simulink model "Complete_Model"  (R2023a)
settings: Solver=VariableStepAuto  StartTime=0.0  StopTime=22  MinStep=5e-5  RelTol=1e-3
values from neighbouring files (symbols this model uses):
  Cbus = 80e-3*n_wt_agg*u^2    (= 1.33333333333)    [param.m]
  D = 1e-3    (= 0.001)    [param.m]
  f = 50    [param.m]
  Fs = Vs * sqrt(2/3)/(2*pi*f)    [param.m]
  fsw = 4e3    (= 4000)    [param.m]
  Idr_rated = sqrt(2)*Is    [param.m]
  J = 127*n_wt_agg    (= 19050)    [param.m]
  ki_id = (wni^2)*Lr*sigma    (= 3.27733001332)    [param.m]
  ki_idg = (wnig^2)*Lg    (= 0.263189450696)    [param.m]
  ki_iq = ki_id    (= 3.27733001332)    [param.m]
  ki_iqg = ki_idg    (= 0.263189450696)    [param.m]
  ki_v = -300000*n_wt_agg    (= -45000000)    [param.m]
  Kopt = ((0.5*rho*pi*(Radius^5)*Cp_max)/(lambda_opt^3))    (= 296454.198186)    [param.m]
  kp_id = (2*wni*sigma*Lr) - Rr    (= 0.00384733333333)    [param.m]
  kp_idg = ((2*wnig*Lg)-Rg)    (= 0.00167538274858)    [param.m]
  kp_iq = kp_id    (= 0.00384733333333)    [param.m]
  kp_iqg = kp_idg    (= 0.00167538274858)    [param.m]
  kp_v = -1000*n_wt_agg    (= -150000)    [param.m]
  Kpg = 1/(1.5*Vr*sqrt(2/3))    [param.m]
  Kqg = -Kpg    [param.m]
  lambda_opt = 7.2    [param.m]
  Lg = 400e-6 /n_wt_agg    (= 2.66666666667e-06)    [param.m]
  Lm = 2.5e-3 /n_wt_agg    (= 1.66666666667e-05)    [param.m]
  Lr = Lm + Lsi    (= 1.72466666667e-05)    [param.m]
  Ls = Lm + Lsi    (= 1.72466666667e-05)    [param.m]
  Lsi = 0.087e-3 /n_wt_agg    (= 5.8e-07)    [param.m]
  N = 100    [param.m]
  n_wt_agg = 150    [param.m]
  p = 2    [param.m]
  Ps = 2e6*n_wt_agg    (= 300000000)    [param.m]
  Radius = 42    [param.m]
  Rg = 20e-6 /n_wt_agg    (= 1.33333333333e-07)    [param.m]
  rho = 1.225    [param.m]
  Rr = 2.9e-3 /n_wt_agg    (= 1.93333333333e-05)    [param.m]
  Rs = 2.6e-3 /n_wt_agg    (= 1.73333333333e-05)    [param.m]
  sigma = 1 - Lm^2 / (Ls*Lr)    (= 0.0661284179513)    [param.m]
  smax = 1/3    (= 0.333333333333)    [param.m]
  tab_lambda = [0.1:0.01:11.8]    [param.m]
  Vbus = 1150/u    (= 3450)    [param.m]
  Vs = 690    [param.m]
undefined symbols (not defined in the file or in its folder): Cp, D1, Delta, DiscIntNeverNeededParam, magnitude
libraries: spsSecondOrderFilterLib x133, spsThreePhaseVIMeasurementLib x58, spsPower3phInstantaneousLib x52, simulink x41, spsThreePhaseTransformerTwoWindingsLib x27, spsUniversalBridgeLib x22, spsPWMGenerator2LevelLib x22, spsSeriesRLCBranchLib x19, spsFirstOrderFilterLib x18, spsThreePhasePISectionLineLib x17, spsGroundLib x17, spsThreePhaseSeriesRLCBranchLib x17
(part 11 of 15)

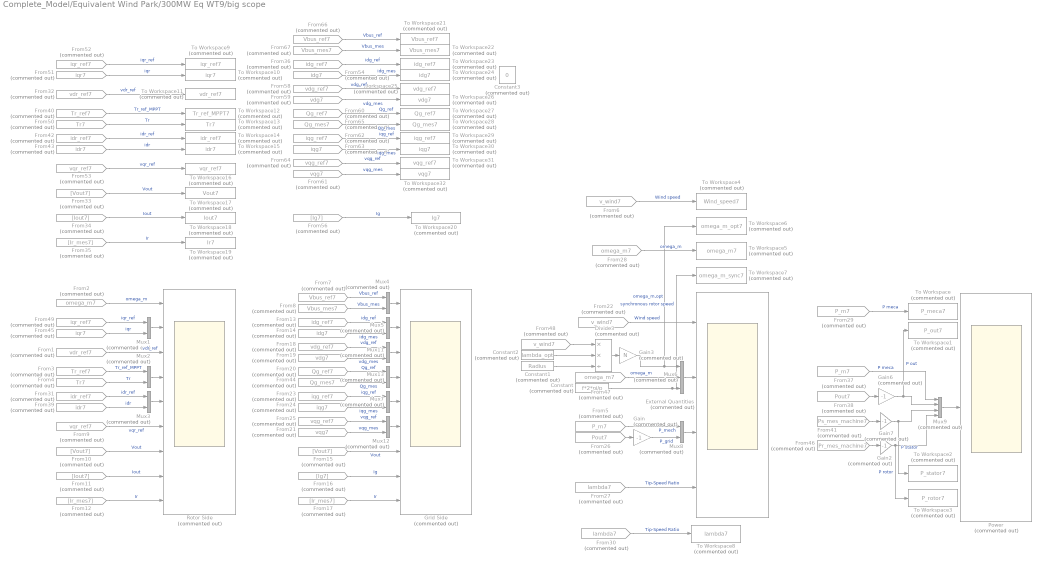
== system Complete_Model/Equivalent Wind Park/300MW Eq WT9/big scope ==
  Constant : Constant  Commented=on  Value=f*2*pi/p
  Constant1 : Constant  Commented=on  Value=Radius
  Constant2 : Constant  Commented=on  Value=lambda_opt
  Constant3 : Constant  Commented=on  Value=0
  Divide3 : Product  Commented=on  Inputs=**/
  External Quantities : Scope  Commented=on  NumInputPorts=4
  From1 : From  Commented=on  GotoTag=vdr_ref7  TagVisibility=global
  From10 : From  Commented=on  GotoTag=Vout7  TagVisibility=global
  From11 : From  Commented=on  GotoTag=Iout7  TagVisibility=global
  From12 : From  Commented=on  GotoTag=Ir_mes7  TagVisibility=global
  From13 : From  Commented=on  GotoTag=idg_ref7  TagVisibility=global
  From14 : From  Commented=on  GotoTag=idg7  TagVisibility=global
  From15 : From  Commented=on  GotoTag=Vout7  TagVisibility=global
  From16 : From  Commented=on  GotoTag=Ig7  TagVisibility=global
  From17 : From  Commented=on  GotoTag=Ir_mes7  TagVisibility=global
  From18 : From  Commented=on  GotoTag=vdg_ref7  TagVisibility=global
  From19 : From  Commented=on  GotoTag=vdg7  TagVisibility=global
  From2 : From  Commented=on  GotoTag=omega_m7  TagVisibility=global
  From20 : From  Commented=on  GotoTag=Qg_ref7  TagVisibility=global
  From21 : From  Commented=on  GotoTag=vqg7  TagVisibility=global
  From22 : From  Commented=on  GotoTag=v_wind7  TagVisibility=global
  From23 : From  Commented=on  GotoTag=iqg_ref7  TagVisibility=global
  From24 : From  Commented=on  GotoTag=iqg7  TagVisibility=global
  From25 : From  Commented=on  GotoTag=vqg_ref7  TagVisibility=global
  From26 : From  Commented=on  GotoTag=Pout7  TagVisibility=global
  From27 : From  Commented=on  GotoTag=lambda7  TagVisibility=global
  From28 : From  Commented=on  GotoTag=omega_m7  TagVisibility=global
  From29 : From  Commented=on  GotoTag=P_m7  TagVisibility=global
  From3 : From  Commented=on  GotoTag=Tr_ref7  TagVisibility=global
  From30 : From  Commented=on  GotoTag=lambda7  TagVisibility=global
  From31 : From  Commented=on  GotoTag=idr_ref7  TagVisibility=global
  From32 : From  Commented=on  GotoTag=vdr_ref7  TagVisibility=global
  From33 : From  Commented=on  GotoTag=Vout7  TagVisibility=global
  From34 : From  Commented=on  GotoTag=Iout7  TagVisibility=global
  From35 : From  Commented=on  GotoTag=Ir_mes7  TagVisibility=global
  From36 : From  Commented=on  GotoTag=idg_ref7  TagVisibility=global
  From37 : From  Commented=on  GotoTag=P_m7  TagVisibility=global
  From38 : From  Commented=on  GotoTag=Pout7  TagVisibility=global
  From39 : From  Commented=on  GotoTag=idr7  TagVisibility=global
  From4 : From  Commented=on  GotoTag=Tr7  TagVisibility=global
  From40 : From  Commented=on  GotoTag=Tr_ref7  TagVisibility=global
  From41 : From  Commented=on  GotoTag=Ps_mes_machine7  TagVisibility=global
  From42 : From  Commented=on  GotoTag=idr_ref7  TagVisibility=global
  From43 : From  Commented=on  GotoTag=idr7  TagVisibility=global
  From44 : From  Commented=on  GotoTag=Qg_mes7  TagVisibility=global
  From45 : From  Commented=on  GotoTag=iqr7  TagVisibility=global
  From46 : From  Commented=on  GotoTag=Pr_mes_machine7  TagVisibility=global
  From47 : From  Commented=on  GotoTag=omega_m7  TagVisibility=global
  From48 : From  Commented=on  GotoTag=v_wind7  TagVisibility=global
  From49 : From  Commented=on  GotoTag=iqr_ref7  TagVisibility=global
  From5 : From  Commented=on  GotoTag=P_m7  TagVisibility=global
  From50 : From  Commented=on  GotoTag=Tr7  TagVisibility=global
  From51 : From  Commented=on  GotoTag=iqr7  TagVisibility=global
  From52 : From  Commented=on  GotoTag=iqr_ref7  TagVisibility=global
  From53 : From  Commented=on  GotoTag=vqr_ref7  TagVisibility=global
  From54 : From  Commented=on  GotoTag=idg7  TagVisibility=global
  From56 : From  Commented=on  GotoTag=Ig7  TagVisibility=global
  From58 : From  Commented=on  GotoTag=vdg_ref7  TagVisibility=global
  From59 : From  Commented=on  GotoTag=vdg7  TagVisibility=global
  From6 : From  Commented=on  GotoTag=v_wind7  TagVisibility=global
  From60 : From  Commented=on  GotoTag=Qg_ref7  TagVisibility=global
  From61 : From  Commented=on  GotoTag=vqg7  TagVisibility=global
  From62 : From  Commented=on  GotoTag=iqg_ref7  TagVisibility=global
  From63 : From  Commented=on  GotoTag=iqg7  TagVisibility=global
  From64 : From  Commented=on  GotoTag=vqg_ref7  TagVisibility=global
  From65 : From  Commented=on  GotoTag=Qg_mes7  TagVisibility=global
  From66 : From  Commented=on  GotoTag=Vbus_ref7  TagVisibility=global
  From67 : From  Commented=on  GotoTag=Vbus_mes7  TagVisibility=global
  From7 : From  Commented=on  GotoTag=Vbus_ref7  TagVisibility=global
  From8 : From  Commented=on  GotoTag=Vbus_mes7  TagVisibility=global
  From9 : From  Commented=on  GotoTag=vqr_ref7  TagVisibility=global
  Gain{,2,6..7} (4 alike) : Gain  Commented=on  Gain=-1
  Gain3 : Gain  Commented=on  Gain=N
  Grid Side : Scope  Commented=on  NumInputPorts=9
  Mux{1..5,7..8,11..13} (10 alike) : Mux  Commented=on  Inputs=2
  Mux6 : Mux  Commented=on  Inputs=3
  Mux9 : Mux  Commented=on
  Power : Scope  Commented=on
  Rotor Side : Scope  Commented=on  NumInputPorts=9
  To Workspace : ToWorkspace  Commented=on  VariableName=P_meca7  SaveFormat=Timeseries  SampleTime=-1
  To Workspace1 : ToWorkspace  Commented=on  VariableName=P_out7  SaveFormat=Timeseries  SampleTime=-1
  To Workspace10 : ToWorkspace  Commented=on  VariableName=iqr7  SaveFormat=Timeseries  SampleTime=-1
  To Workspace11 : ToWorkspace  Commented=on  VariableName=vdr_ref7  SaveFormat=Timeseries  SampleTime=-1
  To Workspace12 : ToWorkspace  Commented=on  VariableName=Tr_ref_MPPT7  SaveFormat=Timeseries  SampleTime=-1
  To Workspace13 : ToWorkspace  Commented=on  VariableName=Tr7  SaveFormat=Timeseries  SampleTime=-1
  To Workspace14 : ToWorkspace  Commented=on  VariableName=idr_ref7  SaveFormat=Timeseries  SampleTime=-1
  To Workspace15 : ToWorkspace  Commented=on  VariableName=idr7  SaveFormat=Timeseries  SampleTime=-1
  To Workspace16 : ToWorkspace  Commented=on  VariableName=vqr_ref7  SaveFormat=Timeseries  SampleTime=-1
  To Workspace17 : ToWorkspace  Commented=on  VariableName=Vout7  SaveFormat=Timeseries  SampleTime=-1
  To Workspace18 : ToWorkspace  Commented=on  VariableName=Iout7  SaveFormat=Timeseries  SampleTime=-1
  To Workspace19 : ToWorkspace  Commented=on  VariableName=Ir7  SaveFormat=Timeseries  SampleTime=-1
  To Workspace2 : ToWorkspace  Commented=on  VariableName=P_stator7  SaveFormat=Timeseries  SampleTime=-1
  To Workspace20 : ToWorkspace  Commented=on  VariableName=Ig7  SaveFormat=Timeseries  SampleTime=-1
  To Workspace21 : ToWorkspace  Commented=on  VariableName=Vbus_ref7  SaveFormat=Timeseries  SampleTime=-1
  To Workspace22 : ToWorkspace  Commented=on  VariableName=Vbus_mes7  SaveFormat=Timeseries  SampleTime=-1
  To Workspace23 : ToWorkspace  Commented=on  VariableName=idg_ref7  SaveFormat=Timeseries  SampleTime=-1
  To Workspace24 : ToWorkspace  Commented=on  VariableName=idg7  SaveFormat=Timeseries  SampleTime=-1
  To Workspace25 : ToWorkspace  Commented=on  VariableName=vdg_ref7  SaveFormat=Timeseries  SampleTime=-1
  To Workspace26 : ToWorkspace  Commented=on  VariableName=vdg7  SaveFormat=Timeseries  SampleTime=-1
  To Workspace27 : ToWorkspace  Commented=on  VariableName=Qg_ref7  SaveFormat=Timeseries  SampleTime=-1
  To Workspace28 : ToWorkspace  Commented=on  VariableName=Qg_mes7  SaveFormat=Timeseries  SampleTime=-1
  To Workspace29 : ToWorkspace  Commented=on  VariableName=iqg_ref7  SaveFormat=Timeseries  SampleTime=-1
  To Workspace3 : ToWorkspace  Commented=on  VariableName=P_rotor7  SaveFormat=Timeseries  SampleTime=-1
  To Workspace30 : ToWorkspace  Commented=on  VariableName=iqg7  SaveFormat=Timeseries  SampleTime=-1
  To Workspace31 : ToWorkspace  Commented=on  VariableName=vqg_ref7  SaveFormat=Timeseries  SampleTime=-1
  To Workspace32 : ToWorkspace  Commented=on  VariableName=vqg7  SaveFormat=Timeseries  SampleTime=-1
  To Workspace4 : ToWorkspace  Commented=on  VariableName=Wind_speed7  SaveFormat=Timeseries  SampleTime=-1
  To Workspace5 : ToWorkspace  Commented=on  VariableName=omega_m7  SaveFormat=Timeseries  SampleTime=-1
  To Workspace6 : ToWorkspace  Commented=on  VariableName=omega_m_opt7  SaveFormat=Timeseries  SampleTime=-1
  To Workspace7 : ToWorkspace  Commented=on  VariableName=omega_m_sync7  SaveFormat=Timeseries  SampleTime=-1
  To Workspace8 : ToWorkspace  Commented=on  VariableName=lambda7  SaveFormat=Timeseries  SampleTime=-1
  To Workspace9 : ToWorkspace  Commented=on  VariableName=iqr_ref7  SaveFormat=Timeseries  SampleTime=-1
  wires:
  From61/1 -> To Workspace32/1  "vqg_mes"
  From64/1 -> To Workspace31/1  "vqg_ref"
  From63/1 -> To Workspace30/1  "iqg_mes"
  From62/1 -> To Workspace29/1  "iqg_ref"
  From65/1 -> To Workspace28/1  "Qg_mes"
  From59/1 -> To Workspace26/1  "vdg_mes"
  From60/1 -> To Workspace27/1  "Qg_ref"
  From58/1 -> To Workspace25/1  "vdg_ref"
  From54/1 -> To Workspace24/1  "idg_mes"
  From36/1 -> To Workspace23/1  "idg_ref"
  From67/1 -> To Workspace22/1  "Vbus_mes"
  From66/1 -> To Workspace21/1  "Vbus_ref"
  From56/1 -> To Workspace20/1  "Ig"
  From35/1 -> To Workspace19/1  "Ir"
  From34/1 -> To Workspace18/1  "Iout"
  From33/1 -> To Workspace17/1  "Vout"
  From53/1 -> To Workspace16/1  "vqr_ref"
  From43/1 -> To Workspace15/1  "idr"
  From42/1 -> To Workspace14/1  "idr_ref"
  From50/1 -> To Workspace13/1  "Tr"
  From40/1 -> To Workspace12/1  "Tr_ref_MPPT"
  From32/1 -> To Workspace11/1  "vdr_ref"
  From51/1 -> To Workspace10/1  "iqr"
  From52/1 -> To Workspace9/1  "iqr_ref"
  From30/1 -> To Workspace8/1  "Tip-Speed Ratio"
  From29/1 -> To Workspace/1  "P meca"
  From28/1 -> To Workspace5/1  "omega_m"
  From6/1 -> To Workspace4/1  "Wind speed"
  From27/1 -> External Quantities/4  "Tip-Speed Ratio"
  Gain3/1 -> Mux6/1, To Workspace6/1  "omega_m,opt"
  Divide3/1 -> Gain3/1
  Constant1/1 -> Divide3/3
  Constant2/1 -> Divide3/2
  From48/1 -> Divide3/1
  From39/1 -> Mux3/2  "idr"
  From4/1 -> Mux2/2  "Tr"
  From1/1 -> Rotor Side/3  "vdr_ref"
  From49/1 -> Mux1/1  "iqr_ref"
  Mux13/1 -> Grid Side/4
  From44/1 -> Mux13/2  "Qg_mes"
  From20/1 -> Mux13/1  "Qg_ref"
  Mux12/1 -> Grid Side/6
  From25/1 -> Mux12/1  "vqg_ref"
  From21/1 -> Mux12/2  "vqg_mes"
  Gain2/1 -> Mux9/4, To Workspace3/1  "P rotor"
  From38/1 -> Gain6/1
  Mux9/1 -> Power/1
  From37/1 -> Mux9/1  "P meca"
  From46/1 -> Gain2/1
  Gain7/1 -> Mux9/3, To Workspace2/1  "P stator"
  From41/1 -> Gain7/1
  Gain6/1 -> Mux9/2, To Workspace1/1  "P out"
  Mux11/1 -> Grid Side/3
  From19/1 -> Mux11/2  "vdg_mes"
  From18/1 -> Mux11/1  "vdg_ref"
  From5/1 -> Mux8/1  "P_mech"
  Constant/1 -> Mux6/3, To Workspace7/1  "synchronous rotor speed"
  From47/1 -> Mux6/2  "omega_m"
  From22/1 -> External Quantities/1  "Wind speed"
  Mux6/1 -> External Quantities/2
  Mux8/1 -> External Quantities/3
  From26/1 -> Gain/1
  Gain/1 -> Mux8/2  "P_grid"
  From2/1 -> Rotor Side/1  "omega_m"
  From13/1 -> Mux5/1  "idg_ref"
  Mux4/1 -> Grid Side/1
  From14/1 -> Mux5/2  "idg_mes"
  Mux5/1 -> Grid Side/2
  From8/1 -> Mux4/2  "Vbus_mes"
  Mux7/1 -> Grid Side/5
  From7/1 -> Mux4/1  "Vbus_ref"
  From24/1 -> Mux7/2  "iqg_mes"
  From17/1 -> Grid Side/9  "Ir"
  From15/1 -> Grid Side/7  "Vout"
  From23/1 -> Mux7/1  "iqg_ref"
  From16/1 -> Grid Side/8  "Ig"
  From12/1 -> Rotor Side/9  "Ir"
  From11/1 -> Rotor Side/8  "Iout"
  From10/1 -> Rotor Side/7  "Vout"
  From9/1 -> Rotor Side/6  "vqr_ref"
  From31/1 -> Mux3/1  "idr_ref"
  Mux3/1 -> Rotor Side/5
  Mux2/1 -> Rotor Side/4
  From3/1 -> Mux2/1  "Tr_ref_MPPT"
  Mux1/1 -> Rotor Side/2
  From45/1 -> Mux1/2  "iqr"

== system Complete_Model/HVDC Link/Grid side control/abc->alpha//beta ==
  abc : Inport
  Fcn1 : Fcn  Expr=2/3 * (u[1]-u[2]/2-u[3]/2)
  Fcn2 : Fcn  Expr=2/3 * (u[2] * (sqrt(3)/2) - u[3]*(sqrt(3)/2))
  Mux : Mux  Inputs=2
  alpha/beta : Outport
  wires:
  abc/1 -> Fcn2/1, Fcn1/1
  Mux/1 -> alpha/beta/1
  Fcn1/1 -> Mux/1
  Fcn2/1 -> Mux/2

== system Complete_Model/HVDC Link/Subsystem7/If Action Subsystem ==
  In1 : Inport
  Action Port : ActionPort  ActionPortLabel=if(u1 <= 1)
  Pref_hvdc : Outport
  wires:
  In1/1 -> Pref_hvdc/1

== system Complete_Model/HVDC Link/Subsystem7/If Action Subsystem 2 ==
  In1 : Inport
  Action Port : ActionPort  ActionPortLabel=else
  Out1 : Outport  VectorParamsAs1DForOutWhenUnconnected=off
  wires:
  In1/1 -> Out1/1

== system Complete_Model/HVDC Link/Wind farm side control/PI_regulator 1 ==
  note: Regulator PI
      | (Type 2)
  Ord : Inport
  Meas : Inport  Port=2
  Add : Sum
  Add1 : Sum
  Discrete-Time Integrator : DiscreteIntegrator  IntegratorMethod=Integration: Trapezoidal  gainval=Ki  InitialCondition=i_ic  SampleTime=Ts  LimitOutput=on  UpperSaturationLimit=max_i  LowerSaturationLimit=min_i  ICPrevOutput=DiscIntNeverNeededParam  ICPrevScaledInput=DiscIntNeverNeededParam
      settings: AttributesFormatString=Gain=%<gainval>\nMin=%<LowerSaturationLimit>\nMax=%<UpperSaturationLimit>
  Kf : Gain  Gain=Kf
      settings: AttributesFormatString=Gain=%<Gain>
  Kp : Gain  Gain=Kp
      settings: AttributesFormatString=Gain=%<Gain>
  Saturation : Saturate  UpperLimit=max_out  LowerLimit=min_out
      settings: AttributesFormatString=Min=%<LowerLimit>\nMax=%<UpperLimit>
  Subtract : Sum  Inputs=+-
  Sum2 : Sum  Inputs=|+-  SaturateOnIntegerOverflow=on
  Unit Delay : UnitDelay  SampleTime=7.4074e-05  HasFrameUpgradeWarning=on
      settings: AttributesFormatString=IC=%<InitialCondition>
  Out : Outport  VectorParamsAs1DForOutWhenUnconnected=off
  wires:
  Meas/1 -> Subtract/2
  Discrete-Time Integrator/1 -> Add1/2
  Add1/1 -> Sum2/2, Saturation/1
  Kf/1 -> Unit Delay/1
  Ord/1 -> Subtract/1
  Add/1 -> Discrete-Time Integrator/1
  Unit Delay/1 -> Add/2
  Saturation/1 -> Out/1, Sum2/1
  Subtract/1 -> Kp/1, Add/1
  Sum2/1 -> Kf/1
  Kp/1 -> Add1/1

== system Complete_Model/HVDC Link/Wind farm side control/PI_regulator 2 ==
  note: Regulator PI
      | (Type 2)
  Ord : Inport
  Meas : Inport  Port=2
  Add : Sum
  Add1 : Sum
  Discrete-Time Integrator : DiscreteIntegrator  IntegratorMethod=Integration: Trapezoidal  gainval=Ki  InitialCondition=i_ic  SampleTime=Ts  LimitOutput=on  UpperSaturationLimit=max_i  LowerSaturationLimit=min_i  ICPrevOutput=DiscIntNeverNeededParam  ICPrevScaledInput=DiscIntNeverNeededParam
      settings: AttributesFormatString=Gain=%<gainval>\nMin=%<LowerSaturationLimit>\nMax=%<UpperSaturationLimit>
  Kf : Gain  Gain=Kf
      settings: AttributesFormatString=Gain=%<Gain>
  Kp : Gain  Gain=Kp
      settings: AttributesFormatString=Gain=%<Gain>
  Saturation : Saturate  UpperLimit=max_out  LowerLimit=min_out
      settings: AttributesFormatString=Min=%<LowerLimit>\nMax=%<UpperLimit>
  Subtract : Sum  Inputs=+-
  Sum2 : Sum  Inputs=|+-  SaturateOnIntegerOverflow=on
  Unit Delay : UnitDelay  SampleTime=7.4074e-05  HasFrameUpgradeWarning=on
      settings: AttributesFormatString=IC=%<InitialCondition>
  Out : Outport  VectorParamsAs1DForOutWhenUnconnected=off
  wires:
  Meas/1 -> Subtract/2
  Discrete-Time Integrator/1 -> Add1/2
  Add1/1 -> Sum2/2, Saturation/1
  Kf/1 -> Unit Delay/1
  Ord/1 -> Subtract/1
  Add/1 -> Discrete-Time Integrator/1
  Unit Delay/1 -> Add/2
  Saturation/1 -> Out/1, Sum2/1
  Subtract/1 -> Kp/1, Add/1
  Sum2/1 -> Kf/1
  Kp/1 -> Add1/1

== system Complete_Model/HVDC Link/Wind farm side control/abc->alpha//beta Clarke ==
  abc : Inport
  Fcn1 : Fcn  Expr=2/3 * (u[1]-u[2]/2-u[3]/2)
  Fcn2 : Fcn  Expr=2/3 * (u[2] * (sqrt(3)/2) - u[3]*(sqrt(3)/2))
  Mux : Mux  Inputs=2
  alpha/beta : Outport
  wires:
  abc/1 -> Fcn2/1, Fcn1/1
  Mux/1 -> alpha/beta/1
  Fcn1/1 -> Mux/1
  Fcn2/1 -> Mux/2

== system Complete_Model/HVDC Link/Wind farm side control/abc->alpha//beta1 ==
  abc : Inport
  Fcn1 : Fcn  Expr=2/3 * (u[1]-u[2]/2-u[3]/2)
  Fcn2 : Fcn  Expr=2/3 * (u[2] * (sqrt(3)/2) - u[3]*(sqrt(3)/2))
  Mux : Mux  Inputs=2
  alpha/beta : Outport
  wires:
  abc/1 -> Fcn2/1, Fcn1/1
  Mux/1 -> alpha/beta/1
  Fcn1/1 -> Mux/1
  Fcn2/1 -> Mux/2

== system Complete_Model/Equivalent Wind Park/300MW Eq WT1/Grid Side Control/PI  ==
  ref : Inport
  meas : Inport  Port=2
  Discrete Derivative : simulink/Discrete/Discrete Derivative  Commented=on  gainval=1.0  ICPrevScaledInput=0.0  OutMin=[-Ps*smax]  OutMax=[Ps*smax]  OutDataType=fixdt(1, 16)  OutScaling=2^-10  DoSatur=off
  Discrete-Time Integrator : DiscreteIntegrator  SampleTime=1/fsw  LimitOutput=on  UpperSaturationLimit=Ps*smax  LowerSaturationLimit=-Ps*smax  ICPrevOutput=DiscIntNeverNeededParam  ICPrevScaledInput=DiscIntNeverNeededParam
  Gain : Gain  Gain=kp_v
  Gain1 : Gain  Gain=ki_v
  Gain2 : Gain  Commented=on  Gain=ki_v
  PID Controller : slpidlib/PID Controller  Commented=on  Controller=PI  Form=Parallel  TimeDomain=Continuous-time  UseExternalTs=off  SampleTime=-1  IntegratorMethod=Forward Euler  FilterMethod=Forward Euler  ControllerParametersSource=internal  P=kp_v  I=ki_v  UseKiTs=off  D=0  N=100  UseFilter=on  TunerSelectOption=Transfer Function Based (PID Tuner App)  InitialConditionSource=internal  InitialConditionForIntegrator=0  InitialConditionForFilter=0  DifferentiatorICPrevScaledInput=0  ExternalReset=none  TrackingMode=off  Kt=1  LimitOutput=on  SatLimitsSource=internal  UpperSaturationLimit=Ps*smax  LowerSaturationLimit=-Ps*smax  LinearizeAsGain=on  AntiWindupMode=clamping  Kb=1  LimitIntegrator=on  UpperIntegratorSaturationLimit=Ps*smax  LowerIntegratorSaturationLimit=-Ps*smax  POutMin=[]  POutMax=[]  IOutMin=[]  IOutMax=[]  DOutMin=[]  DOutMax=[]  NOutMin=[]  NOutMax=[]  SumOutMin=[]  SumOutMax=[]  SaturationOutMin=[]  SaturationOutMax=[]  PParamMin=[]  PParamMax=[]  IParamMin=[]  IParamMax=[]  DParamMin=[]  DParamMax=[]  NParamMin=[]  NParamMax=[]  KbParamMin=[]  KbParamMax=[]  KtParamMin=[]  KtParamMax=[]  KbOutMin=[]  KbOutMax=[]  KtOutMin=[]  KtOutMax=[]  IntegratorOutMin=[]  IntegratorOutMax=[]  FilterOutMin=[]  FilterOutMax=[]  SumI1OutMin=[]  SumI1OutMax=[]  SumI2OutMin=[]  SumI2OutMax=[]  SumI3OutMin=[]  SumI3OutMax=[]  SumI4OutMin=[]  SumI4OutMax=[]  SumDOutMin=[]  SumDOutMax=[]  DifferentiatorOutMin=[]  DifferentiatorOutMax=[]  FilterDiffOutCoefMin=[]  FilterDiffOutCoefMax=[]  ReciprocalOutMin=[]  ReciprocalOutMax=[]  SumDenOutMin=[]  SumDenOutMax=[]  SumNumOutMin=[]  SumNumOutMax=[]  DivideOutMin=[]  DivideOutMax=[]  UdiffTsProdOutMin=[]  UdiffTsProdOutMax=[]  NTsProdOutMin=[]  NTsProdOutMax=[]  UintegralTsProdOutMin=[]  UintegralTsProdOutMax=[]  UngainTsProdOutMin=[]  UngainTsProdOutMax=[]  ClampingZeroOutDataTypeStr=Inherit: Inherit via back propagation  ClampingZeroOutMin=[]  ClampingZeroOutMax=[]  FilterDenConstantOutDataTypeStr=Inherit: Inherit via back propagation  FilterDenConstantOutMin=[]  FilterDenConstantOutMax=[]  PCopyOutDataTypeStr=Inherit: Inherit via back propagation  PCopyOutMin=[]  PCopyOutMax=[]  NCopyOutDataTypeStr=Inherit: Inherit via back propagation  NCopyOutMin=[]  NCopyOutMax=[]  IntegratorContinuousStateAttributes=''  IntegratorStateMustResolveToSignalObject=off  IntegratorRTWStateStorageClass=Auto  FilterContinuousStateAttributes=''  FilterStateMustResolveToSignalObject=off  FilterRTWStateStorageClass=Auto  ParallelPVariant=InternalParameters  IdealPVariant=Disabled  IVariant=InternalParameters  DVariant=Disabled  IntegratorVariant=Continuous  SatVariant=Enabled  AWVariant=ContClampingParallel  PCopyVariant=Disabled  TRVariant=Disabled  FdbkBlocksVariant=Forward  IdealPFdbkVariant=Disabled  SatFdbkVariant=Disabled  DerivativeFilterVariant=Disabled  NVariant=Disabled  NCopyVariant=Disabled_SignalSpec  FilterICVariant=Disabled  IntegratorICVariant=InternalICs  ExternalResetVariant=Disabled  TRSumVariant=Passthrough  SumFdbkVariant=Disabled  SumVariant=Sum_PI  TsampFilterVariant=InternalTs  TsampNgainVariant=Passthrough  TsampIntegralVariant=Disabled_SignalSpec  ExternalSaturationOutDataTypeStr=Inherit: Same as second input
  Saturation : Saturate  UpperLimit=Ps*smax  LowerLimit=-Ps*smax
  Sum : Sum  Inputs=|+-
  Sum1 : Sum  Inputs=|+++
  out : Outport
  wires:
  Saturation/1 -> out/1
  ref/1 -> Sum/1
  meas/1 -> Sum/2
  Sum/1 -> Gain2/1, Gain1/1, Gain/1
  Gain/1 -> Sum1/1
  Gain1/1 -> Discrete-Time Integrator/1
  Discrete-Time Integrator/1 -> Sum1/2
  Sum1/1 -> Saturation/1
  Gain2/1 -> Discrete Derivative/1
  Discrete Derivative/1 -> Sum1/3

== system Complete_Model/Equivalent Wind Park/300MW Eq WT1/Grid Side Control/PI id ==
  ref : Inport
  meas : Inport  Port=2
  Discrete-Time Integrator : DiscreteIntegrator  SampleTime=1/fsw  LimitOutput=on  UpperSaturationLimit=Vbus/sqrt(3)  LowerSaturationLimit=-Vbus/sqrt(3)  ICPrevOutput=DiscIntNeverNeededParam  ICPrevScaledInput=DiscIntNeverNeededParam
  Gain : Gain  Gain=kp_idg
  Gain1 : Gain  Gain=ki_idg
  Saturation : Saturate  UpperLimit=Vbus/sqrt(3)  LowerLimit=-Vbus/sqrt(3)
  Sum : Sum  Inputs=|+-
  Sum1 : Sum  Inputs=|++
  out : Outport
  wires:
  Saturation/1 -> out/1
  ref/1 -> Sum/1
  meas/1 -> Sum/2
  Sum/1 -> Gain1/1, Gain/1
  Gain/1 -> Sum1/1
  Gain1/1 -> Discrete-Time Integrator/1
  Discrete-Time Integrator/1 -> Sum1/2
  Sum1/1 -> Saturation/1
